simulink model "chapter2_5"  (R2022a)
settings: Solver=FixedStepAuto  StartTime=0.0  StopTime=8  FixedStep=3e-3  RelTol=1e-3
values from neighbouring files (symbols this model uses):
  A = 10    [Figure2_3.m]    (also in parameterLoadForchapter2_4.m = 0.0001; parameterLoadForchapter2_5.m = 0.0001)
  base = Mb*9.8/4+mw*9.8+0.5*9.8*Mv    (= 46305)    [parameterLoadForchapter2_5.m]
  d = 2.6    [parameterLoadForchapter2_4.m]    (also in parameterLoadForchapter2_5.m)
  I = 3055e-8    (= 3.055e-05)    [parameterLoadForchapter2_5.m]
  Jv = 3044    [parameterLoadForchapter2_4.m]    (also in parameterLoadForchapter2_5.m)
  kf = 6.67e7    (= 66700000)    [parameterLoadForchapter2_5.m]
  Kv = 1.5e6    (= 1500000)    [parameterLoadForchapter2_4.m]    (also in parameterLoadForchapter2_5.m)
  L = 15.6    [parameterLoadForchapter2_4.m]    (also in parameterLoadForchapter2_5.m = 210)
  Ln = LNs(i)    [Mchapter2_4.m]    (also in Mchapter2_5.m = LN_set(i))
  m = 7800*(7684e-6)    (= 59.9352)    [parameterLoadForchapter2_5.m]
  Mv = 4060    [parameterLoadForchapter2_4.m]    (also in parameterLoadForchapter2_5.m = 2030)
  mw = 1670    [parameterLoadForchapter2_4.m]    (also in parameterLoadForchapter2_5.m = 835)
  N1 = 0    [Mchapter2_5.m]
  N2 = 0    [Mchapter2_5.m]
  position = 0.5*L    (= 105)    [parameterLoadForchapter2_5.m]
  r = 0.42    [parameterLoadForchapter2_4.m]    (also in parameterLoadForchapter2_5.m)
  R = 400    [Figure2_3.m]    (also in parameterLoadForchapter2_4.m = 0.42; parameterLoadForchapter2_5.m = 0.42)
  v = vkmh/3.6    (= 13.8888888889)    [parameterLoadForchapter2_4.m]    (also in parameterLoadForchapter2_5.m = 27.7777777778)
  vkmh = 50    [parameterLoadForchapter2_4.m]    (also in parameterLoadForchapter2_5.m = 100)
(part 154 of 157)

== system chapter2_5/Subsystem5/Subsystem3/Subsystem6/Subsystem19/Subsystem ==
  In1 : Inport
  k : Inport  Port=2
  In3 : Inport  Port=3
  Add : Sum
  Add1 : Sum  Inputs=-+
  Add2 : Sum  Inputs=+-
  Add3 : Sum  Inputs=+-
  Add4 : Sum  Inputs=+-
  Add5 : Sum  Inputs=-+
  Clock : Clock
  Clock1 : Clock
  Constant : Constant  Value=4
  Constant1 : Constant  Value=kf/m
  Constant2 : Constant
  Constant3 : Constant
  Divide : Product  Inputs=*/
  Divide1 : Product  Inputs=*/
  Divide2 : Product  Inputs=**/
  Gain : Gain  Gain=pi/L
  Gain1 : Gain  Gain=E*I/m
  Gain2 : Gain  Gain=v*pi/L
  Gain3 : Gain  Gain=d/v
  Gain4 : Gain  Gain=-2/(m*L)
  Gain5 : Gain  Gain=pi*position/L
  Power : Math  Operator=pow
  Product{,1..3} (4 alike) : Product
  Sin : Trigonometry
  Sin1 : Trigonometry
  Sin2 : Trigonometry
  Sqrt : Sqrt
  Square : Math  Operator=square
  Square1 : Math  Operator=square
  Out1 : Outport
  tk : Outport  Port=2
  wires:
  [imod] -> Gain/1
  Gain2/1 -> [Omiga_n]
  Gain/1 -> Power/1
  Power/1 -> Gain1/1
  Add/1 -> Sqrt/1
  Gain4/1 -> Divide1/1
  Sin2/1 -> Product2/2
  [Omiga_n] -> Product3/1
  Add3/1 -> Product3/2
  Gain1/1 -> Add/1
  Constant1/1 -> Add/2
  Product3/1 -> Sin1/1
  Sqrt/1 -> [Omiga_bn]
  k/1 -> Add4/1
  Add1/1 -> Divide2/3
  Square/1 -> Add1/1
  Gain5/1 -> Sin/1
  [static_n] -> Product/2
  [Omiga_bn] -> Product1/1
  In1/1 -> [imod]
  Constant2/1 -> Add1/2
  Product/1 -> Divide2/1
  [imod] -> Gain2/1
  Divide/1 -> [Sn]
  [Sn] -> Product2/1
  In3/1 -> Gain4/1
  Divide1/1 -> [static_n]
  [Omiga_bn] -> Square1/1
  [Omiga_n] -> Divide/1
  Square1/1 -> Divide1/2
  [Omiga_bn] -> Divide/2
  [Sn] -> Square/1
  Product2/1 -> Add2/2
  Constant/1 -> Power/2
  [imod] -> Gain5/1
  Sin1/1 -> Add2/1
  Sin/1 -> Product/1
  Product1/1 -> Sin2/1
  Clock/1 -> Add5/2
  Add2/1 -> Divide2/2
  Divide2/1 -> Out1/1
  Clock1/1 -> Add3/1
  Constant3/1 -> Add4/2
  Add4/1 -> Gain3/1
  Gain3/1 -> tk/1, Add5/1, Add3/2
  Add5/1 -> Product1/2

== system chapter2_5/Subsystem5/Subsystem3/Subsystem6/Subsystem19/Subsystem1 ==
  index : Inport
  k : Inport  Port=2
  F : Inport  Port=3
  Add : Sum
  Add1 : Sum  Inputs=-+
  Add2 : Sum  Inputs=+-
  Add3 : Sum  Inputs=+-
  Add4 : Sum  Inputs=+-
  Add5 : Sum  Inputs=-+
  Clock : Clock
  Clock1 : Clock
  Constant : Constant  Value=4
  Constant1 : Constant  Value=kf/m
  Constant2 : Constant
  Constant3 : Constant
  Divide : Product  Inputs=*/
  Divide1 : Product  Inputs=*/
  Divide2 : Product  Inputs=**/
  Gain : Gain  Gain=pi/L
  Gain1 : Gain  Gain=E*I/m
  Gain2 : Gain  Gain=v*pi/L
  Gain3 : Gain  Gain=d/v
  Gain4 : Gain  Gain=-2/(m*L)
  Gain5 : Gain  Gain=pi*position/L
  Power : Math  Operator=pow
  Product{,1..3} (4 alike) : Product
  Sin : Trigonometry
  Sin1 : Trigonometry
  Sin2 : Trigonometry
  Sqrt : Sqrt
  Square : Math  Operator=square
  Square1 : Math  Operator=square
  Out1 : Outport
  tk : Outport  Port=2
  wires:
  Add5/1 -> Product1/2
  Gain3/1 -> Add3/2, Add5/1, tk/1
  Add4/1 -> Gain3/1
  Constant3/1 -> Add4/2
  Clock1/1 -> Add3/1
  Divide2/1 -> Out1/1
  Add2/1 -> Divide2/2
  Clock/1 -> Add5/2
  Product1/1 -> Sin2/1
  Sin/1 -> Product/1
  Sin1/1 -> Add2/1
  [imod] -> Gain5/1
  Constant/1 -> Power/2
  Product2/1 -> Add2/2
  [Sn] -> Square/1
  [Omiga_bn] -> Divide/2
  Square1/1 -> Divide1/2
  [Omiga_n] -> Divide/1
  [Omiga_bn] -> Square1/1
  Divide1/1 -> [static_n]
  F/1 -> Gain4/1
  [Sn] -> Product2/1
  Divide/1 -> [Sn]
  [imod] -> Gain2/1
  Product/1 -> Divide2/1
  Constant2/1 -> Add1/2
  index/1 -> [imod]
  [Omiga_bn] -> Product1/1
  [static_n] -> Product/2
  Gain5/1 -> Sin/1
  Square/1 -> Add1/1
  Add1/1 -> Divide2/3
  k/1 -> Add4/1
  Sqrt/1 -> [Omiga_bn]
  Product3/1 -> Sin1/1
  Constant1/1 -> Add/2
  Gain1/1 -> Add/1
  Add3/1 -> Product3/2
  [Omiga_n] -> Product3/1
  Sin2/1 -> Product2/2
  Gain4/1 -> Divide1/1
  Add/1 -> Sqrt/1
  Power/1 -> Gain1/1
  Gain/1 -> Power/1
  Gain2/1 -> [Omiga_n]
  [imod] -> Gain/1

== system chapter2_5/Subsystem5/Subsystem3/Subsystem6/Subsystem19/Subsystem2 ==
  uxt1 : Inport
  tk : Inport  Port=2
  Add : Sum  Inputs=+-
  Add1 : Sum  Inputs=+--
  Add2 : Sum  Inputs=+-
  C : Constant
  C1 : Constant  Value=0
  C2 : Constant
  C3 : Constant  Value=0
  Clock : Clock
  Clock1 : Clock
  Product : Product
  Switch : Switch  Criteria=u2 > Threshold  SaturateOnIntegerOverflow=off  InputSameDT=off
  Switch1 : Switch  Criteria=u2 > Threshold  SaturateOnIntegerOverflow=off  InputSameDT=off
  deltaT : Constant  Value=L/v
  U1 : Outport
  wires:
  uxt1/1 -> Product/1
  Product/1 -> U1/1
  Clock/1 -> Add/1
  Add/1 -> Switch/2
  C/1 -> Switch/1
  C1/1 -> Switch/3
  C3/1 -> Switch1/3
  C2/1 -> Switch1/1
  Add1/1 -> Switch1/2
  tk/1 -> Add1/2, Add/2
  Clock1/1 -> Add1/1
  deltaT/1 -> Add1/3
  Switch/1 -> Add2/1
  Switch1/1 -> Add2/2
  Add2/1 -> Product/2

== system chapter2_5/Subsystem5/Subsystem3/Subsystem6/Subsystem19/Subsystem3 ==
  uxt1 : Inport
  tk : Inport  Port=2
  Add : Sum  Inputs=+-
  Add1 : Sum  Inputs=+--
  Add2 : Sum  Inputs=+-
  C : Constant
  C1 : Constant  Value=0
  C2 : Constant
  C3 : Constant  Value=0
  Clock : Clock
  Clock1 : Clock
  Product : Product
  Switch : Switch  Criteria=u2 > Threshold  SaturateOnIntegerOverflow=off  InputSameDT=off
  Switch1 : Switch  Criteria=u2 > Threshold  SaturateOnIntegerOverflow=off  InputSameDT=off
  deltaT : Constant  Value=L/v
  U1 : Outport
  wires:
  Add2/1 -> Product/2
  Switch1/1 -> Add2/2
  Switch/1 -> Add2/1
  deltaT/1 -> Add1/3
  Clock1/1 -> Add1/1
  tk/1 -> Add/2, Add1/2
  Add1/1 -> Switch1/2
  C2/1 -> Switch1/1
  C3/1 -> Switch1/3
  C1/1 -> Switch/3
  C/1 -> Switch/1
  Add/1 -> Switch/2
  Clock/1 -> Add/1
  Product/1 -> U1/1
  uxt1/1 -> Product/1

== system chapter2_5/Subsystem5/Subsystem3/Subsystem6/Subsystem2/Subsystem ==
  In1 : Inport
  k : Inport  Port=2
  In3 : Inport  Port=3
  Add : Sum
  Add1 : Sum  Inputs=-+
  Add2 : Sum  Inputs=+-
  Add3 : Sum  Inputs=+-
  Add4 : Sum  Inputs=+-
  Add5 : Sum  Inputs=-+
  Clock : Clock
  Clock1 : Clock
  Constant : Constant  Value=4
  Constant1 : Constant  Value=kf/m
  Constant2 : Constant
  Constant3 : Constant
  Divide : Product  Inputs=*/
  Divide1 : Product  Inputs=*/
  Divide2 : Product  Inputs=**/
  Gain : Gain  Gain=pi/L
  Gain1 : Gain  Gain=E*I/m
  Gain2 : Gain  Gain=v*pi/L
  Gain3 : Gain  Gain=d/v
  Gain4 : Gain  Gain=-2/(m*L)
  Gain5 : Gain  Gain=pi*position/L
  Power : Math  Operator=pow
  Product{,1..3} (4 alike) : Product
  Sin : Trigonometry
  Sin1 : Trigonometry
  Sin2 : Trigonometry
  Sqrt : Sqrt
  Square : Math  Operator=square
  Square1 : Math  Operator=square
  Out1 : Outport
  tk : Outport  Port=2
  wires:
  [imod] -> Gain/1
  Gain2/1 -> [Omiga_n]
  Gain/1 -> Power/1
  Power/1 -> Gain1/1
  Add/1 -> Sqrt/1
  Gain4/1 -> Divide1/1
  Sin2/1 -> Product2/2
  [Omiga_n] -> Product3/1
  Add3/1 -> Product3/2
  Gain1/1 -> Add/1
  Constant1/1 -> Add/2
  Product3/1 -> Sin1/1
  Sqrt/1 -> [Omiga_bn]
  k/1 -> Add4/1
  Add1/1 -> Divide2/3
  Square/1 -> Add1/1
  Gain5/1 -> Sin/1
  [static_n] -> Product/2
  [Omiga_bn] -> Product1/1
  In1/1 -> [imod]
  Constant2/1 -> Add1/2
  Product/1 -> Divide2/1
  [imod] -> Gain2/1
  Divide/1 -> [Sn]
  [Sn] -> Product2/1
  In3/1 -> Gain4/1
  Divide1/1 -> [static_n]
  [Omiga_bn] -> Square1/1
  [Omiga_n] -> Divide/1
  Square1/1 -> Divide1/2
  [Omiga_bn] -> Divide/2
  [Sn] -> Square/1
  Product2/1 -> Add2/2
  Constant/1 -> Power/2
  [imod] -> Gain5/1
  Sin1/1 -> Add2/1
  Sin/1 -> Product/1
  Product1/1 -> Sin2/1
  Clock/1 -> Add5/2
  Add2/1 -> Divide2/2
  Divide2/1 -> Out1/1
  Clock1/1 -> Add3/1
  Constant3/1 -> Add4/2
  Add4/1 -> Gain3/1
  Gain3/1 -> tk/1, Add5/1, Add3/2
  Add5/1 -> Product1/2

== system chapter2_5/Subsystem5/Subsystem3/Subsystem6/Subsystem2/Subsystem1 ==
  index : Inport
  k : Inport  Port=2
  F : Inport  Port=3
  Add : Sum
  Add1 : Sum  Inputs=-+
  Add2 : Sum  Inputs=+-
  Add3 : Sum  Inputs=+-
  Add4 : Sum  Inputs=+-
  Add5 : Sum  Inputs=-+
  Clock : Clock
  Clock1 : Clock
  Constant : Constant  Value=4
  Constant1 : Constant  Value=kf/m
  Constant2 : Constant
  Constant3 : Constant
  Divide : Product  Inputs=*/
  Divide1 : Product  Inputs=*/
  Divide2 : Product  Inputs=**/
  Gain : Gain  Gain=pi/L
  Gain1 : Gain  Gain=E*I/m
  Gain2 : Gain  Gain=v*pi/L
  Gain3 : Gain  Gain=d/v
  Gain4 : Gain  Gain=-2/(m*L)
  Gain5 : Gain  Gain=pi*position/L
  Power : Math  Operator=pow
  Product{,1..3} (4 alike) : Product
  Sin : Trigonometry
  Sin1 : Trigonometry
  Sin2 : Trigonometry
  Sqrt : Sqrt
  Square : Math  Operator=square
  Square1 : Math  Operator=square
  Out1 : Outport
  tk : Outport  Port=2
  wires:
  Add5/1 -> Product1/2
  Gain3/1 -> Add3/2, Add5/1, tk/1
  Add4/1 -> Gain3/1
  Constant3/1 -> Add4/2
  Clock1/1 -> Add3/1
  Divide2/1 -> Out1/1
  Add2/1 -> Divide2/2
  Clock/1 -> Add5/2
  Product1/1 -> Sin2/1
  Sin/1 -> Product/1
  Sin1/1 -> Add2/1
  [imod] -> Gain5/1
  Constant/1 -> Power/2
  Product2/1 -> Add2/2
  [Sn] -> Square/1
  [Omiga_bn] -> Divide/2
  Square1/1 -> Divide1/2
  [Omiga_n] -> Divide/1
  [Omiga_bn] -> Square1/1
  Divide1/1 -> [static_n]
  F/1 -> Gain4/1
  [Sn] -> Product2/1
  Divide/1 -> [Sn]
  [imod] -> Gain2/1
  Product/1 -> Divide2/1
  Constant2/1 -> Add1/2
  index/1 -> [imod]
  [Omiga_bn] -> Product1/1
  [static_n] -> Product/2
  Gain5/1 -> Sin/1
  Square/1 -> Add1/1
  Add1/1 -> Divide2/3
  k/1 -> Add4/1
  Sqrt/1 -> [Omiga_bn]
  Product3/1 -> Sin1/1
  Constant1/1 -> Add/2
  Gain1/1 -> Add/1
  Add3/1 -> Product3/2
  [Omiga_n] -> Product3/1
  Sin2/1 -> Product2/2
  Gain4/1 -> Divide1/1
  Add/1 -> Sqrt/1
  Power/1 -> Gain1/1
  Gain/1 -> Power/1
  Gain2/1 -> [Omiga_n]
  [imod] -> Gain/1

== system chapter2_5/Subsystem5/Subsystem3/Subsystem6/Subsystem2/Subsystem2 ==
  uxt1 : Inport
  tk : Inport  Port=2
  Add : Sum  Inputs=+-
  Add1 : Sum  Inputs=+--
  Add2 : Sum  Inputs=+-
  C : Constant
  C1 : Constant  Value=0
  C2 : Constant
  C3 : Constant  Value=0
  Clock : Clock
  Clock1 : Clock
  Product : Product
  Switch : Switch  Criteria=u2 > Threshold  SaturateOnIntegerOverflow=off  InputSameDT=off
  Switch1 : Switch  Criteria=u2 > Threshold  SaturateOnIntegerOverflow=off  InputSameDT=off
  deltaT : Constant  Value=L/v
  U1 : Outport
  wires:
  uxt1/1 -> Product/1
  Product/1 -> U1/1
  Clock/1 -> Add/1
  Add/1 -> Switch/2
  C/1 -> Switch/1
  C1/1 -> Switch/3
  C3/1 -> Switch1/3
  C2/1 -> Switch1/1
  Add1/1 -> Switch1/2
  tk/1 -> Add1/2, Add/2
  Clock1/1 -> Add1/1
  deltaT/1 -> Add1/3
  Switch/1 -> Add2/1
  Switch1/1 -> Add2/2
  Add2/1 -> Product/2

== system chapter2_5/Subsystem5/Subsystem3/Subsystem6/Subsystem2/Subsystem3 ==
  uxt1 : Inport
  tk : Inport  Port=2
  Add : Sum  Inputs=+-
  Add1 : Sum  Inputs=+--
  Add2 : Sum  Inputs=+-
  C : Constant
  C1 : Constant  Value=0
  C2 : Constant
  C3 : Constant  Value=0
  Clock : Clock
  Clock1 : Clock
  Product : Product
  Switch : Switch  Criteria=u2 > Threshold  SaturateOnIntegerOverflow=off  InputSameDT=off
  Switch1 : Switch  Criteria=u2 > Threshold  SaturateOnIntegerOverflow=off  InputSameDT=off
  deltaT : Constant  Value=L/v
  U1 : Outport
  wires:
  Add2/1 -> Product/2
  Switch1/1 -> Add2/2
  Switch/1 -> Add2/1
  deltaT/1 -> Add1/3
  Clock1/1 -> Add1/1
  tk/1 -> Add/2, Add1/2
  Add1/1 -> Switch1/2
  C2/1 -> Switch1/1
  C3/1 -> Switch1/3
  C1/1 -> Switch/3
  C/1 -> Switch/1
  Add/1 -> Switch/2
  Clock/1 -> Add/1
  Product/1 -> U1/1
  uxt1/1 -> Product/1

== system chapter2_5/Subsystem5/Subsystem3/Subsystem6/Subsystem21/Subsystem ==
  In1 : Inport
  k : Inport  Port=2
  In3 : Inport  Port=3
  Add : Sum
  Add1 : Sum  Inputs=-+
  Add2 : Sum  Inputs=+-
  Add3 : Sum  Inputs=+-
  Add4 : Sum  Inputs=+-
  Add5 : Sum  Inputs=-+
  Clock : Clock
  Clock1 : Clock
  Constant : Constant  Value=4
  Constant1 : Constant  Value=kf/m
  Constant2 : Constant
  Constant3 : Constant
  Divide : Product  Inputs=*/
  Divide1 : Product  Inputs=*/
  Divide2 : Product  Inputs=**/
  Gain : Gain  Gain=pi/L
  Gain1 : Gain  Gain=E*I/m
  Gain2 : Gain  Gain=v*pi/L
  Gain3 : Gain  Gain=d/v
  Gain4 : Gain  Gain=-2/(m*L)
  Gain5 : Gain  Gain=pi*position/L
  Power : Math  Operator=pow
  Product{,1..3} (4 alike) : Product
  Sin : Trigonometry
  Sin1 : Trigonometry
  Sin2 : Trigonometry
  Sqrt : Sqrt
  Square : Math  Operator=square
  Square1 : Math  Operator=square
  Out1 : Outport
  tk : Outport  Port=2
  wires:
  [imod] -> Gain/1
  Gain2/1 -> [Omiga_n]
  Gain/1 -> Power/1
  Power/1 -> Gain1/1
  Add/1 -> Sqrt/1
  Gain4/1 -> Divide1/1
  Sin2/1 -> Product2/2
  [Omiga_n] -> Product3/1
  Add3/1 -> Product3/2
  Gain1/1 -> Add/1
  Constant1/1 -> Add/2
  Product3/1 -> Sin1/1
  Sqrt/1 -> [Omiga_bn]
  k/1 -> Add4/1
  Add1/1 -> Divide2/3
  Square/1 -> Add1/1
  Gain5/1 -> Sin/1
  [static_n] -> Product/2
  [Omiga_bn] -> Product1/1
  In1/1 -> [imod]
  Constant2/1 -> Add1/2
  Product/1 -> Divide2/1
  [imod] -> Gain2/1
  Divide/1 -> [Sn]
  [Sn] -> Product2/1
  In3/1 -> Gain4/1
  Divide1/1 -> [static_n]
  [Omiga_bn] -> Square1/1
  [Omiga_n] -> Divide/1
  Square1/1 -> Divide1/2
  [Omiga_bn] -> Divide/2
  [Sn] -> Square/1
  Product2/1 -> Add2/2
  Constant/1 -> Power/2
  [imod] -> Gain5/1
  Sin1/1 -> Add2/1
  Sin/1 -> Product/1
  Product1/1 -> Sin2/1
  Clock/1 -> Add5/2
  Add2/1 -> Divide2/2
  Divide2/1 -> Out1/1
  Clock1/1 -> Add3/1
  Constant3/1 -> Add4/2
  Add4/1 -> Gain3/1
  Gain3/1 -> tk/1, Add5/1, Add3/2
  Add5/1 -> Product1/2

== system chapter2_5/Subsystem5/Subsystem3/Subsystem6/Subsystem21/Subsystem1 ==
  index : Inport
  k : Inport  Port=2
  F : Inport  Port=3
  Add : Sum
  Add1 : Sum  Inputs=-+
  Add2 : Sum  Inputs=+-
  Add3 : Sum  Inputs=+-
  Add4 : Sum  Inputs=+-
  Add5 : Sum  Inputs=-+
  Clock : Clock
  Clock1 : Clock
  Constant : Constant  Value=4
  Constant1 : Constant  Value=kf/m
  Constant2 : Constant
  Constant3 : Constant
  Divide : Product  Inputs=*/
  Divide1 : Product  Inputs=*/
  Divide2 : Product  Inputs=**/
  Gain : Gain  Gain=pi/L
  Gain1 : Gain  Gain=E*I/m
  Gain2 : Gain  Gain=v*pi/L
  Gain3 : Gain  Gain=d/v
  Gain4 : Gain  Gain=-2/(m*L)
  Gain5 : Gain  Gain=pi*position/L
  Power : Math  Operator=pow
  Product{,1..3} (4 alike) : Product
  Sin : Trigonometry
  Sin1 : Trigonometry
  Sin2 : Trigonometry
  Sqrt : Sqrt
  Square : Math  Operator=square
  Square1 : Math  Operator=square
  Out1 : Outport
  tk : Outport  Port=2
  wires:
  Add5/1 -> Product1/2
  Gain3/1 -> Add3/2, Add5/1, tk/1
  Add4/1 -> Gain3/1
  Constant3/1 -> Add4/2
  Clock1/1 -> Add3/1
  Divide2/1 -> Out1/1
  Add2/1 -> Divide2/2
  Clock/1 -> Add5/2
  Product1/1 -> Sin2/1
  Sin/1 -> Product/1
  Sin1/1 -> Add2/1
  [imod] -> Gain5/1
  Constant/1 -> Power/2
  Product2/1 -> Add2/2
  [Sn] -> Square/1
  [Omiga_bn] -> Divide/2
  Square1/1 -> Divide1/2
  [Omiga_n] -> Divide/1
  [Omiga_bn] -> Square1/1
  Divide1/1 -> [static_n]
  F/1 -> Gain4/1
  [Sn] -> Product2/1
  Divide/1 -> [Sn]
  [imod] -> Gain2/1
  Product/1 -> Divide2/1
  Constant2/1 -> Add1/2
  index/1 -> [imod]
  [Omiga_bn] -> Product1/1
  [static_n] -> Product/2
  Gain5/1 -> Sin/1
  Square/1 -> Add1/1
  Add1/1 -> Divide2/3
  k/1 -> Add4/1
  Sqrt/1 -> [Omiga_bn]
  Product3/1 -> Sin1/1
  Constant1/1 -> Add/2
  Gain1/1 -> Add/1
  Add3/1 -> Product3/2
  [Omiga_n] -> Product3/1
  Sin2/1 -> Product2/2
  Gain4/1 -> Divide1/1
  Add/1 -> Sqrt/1
  Power/1 -> Gain1/1
  Gain/1 -> Power/1
  Gain2/1 -> [Omiga_n]
  [imod] -> Gain/1

== system chapter2_5/Subsystem5/Subsystem3/Subsystem6/Subsystem21/Subsystem2 ==
  uxt1 : Inport
  tk : Inport  Port=2
  Add : Sum  Inputs=+-
  Add1 : Sum  Inputs=+--
  Add2 : Sum  Inputs=+-
  C : Constant
  C1 : Constant  Value=0
  C2 : Constant
  C3 : Constant  Value=0
  Clock : Clock
  Clock1 : Clock
  Product : Product
  Switch : Switch  Criteria=u2 > Threshold  SaturateOnIntegerOverflow=off  InputSameDT=off
  Switch1 : Switch  Criteria=u2 > Threshold  SaturateOnIntegerOverflow=off  InputSameDT=off
  deltaT : Constant  Value=L/v
  U1 : Outport
  wires:
  uxt1/1 -> Product/1
  Product/1 -> U1/1
  Clock/1 -> Add/1
  Add/1 -> Switch/2
  C/1 -> Switch/1
  C1/1 -> Switch/3
  C3/1 -> Switch1/3
  C2/1 -> Switch1/1
  Add1/1 -> Switch1/2
  tk/1 -> Add1/2, Add/2
  Clock1/1 -> Add1/1
  deltaT/1 -> Add1/3
  Switch/1 -> Add2/1
  Switch1/1 -> Add2/2
  Add2/1 -> Product/2

== system chapter2_5/Subsystem5/Subsystem3/Subsystem6/Subsystem21/Subsystem3 ==
  uxt1 : Inport
  tk : Inport  Port=2
  Add : Sum  Inputs=+-
  Add1 : Sum  Inputs=+--
  Add2 : Sum  Inputs=+-
  C : Constant
  C1 : Constant  Value=0
  C2 : Constant
  C3 : Constant  Value=0
  Clock : Clock
  Clock1 : Clock
  Product : Product
  Switch : Switch  Criteria=u2 > Threshold  SaturateOnIntegerOverflow=off  InputSameDT=off
  Switch1 : Switch  Criteria=u2 > Threshold  SaturateOnIntegerOverflow=off  InputSameDT=off
  deltaT : Constant  Value=L/v
  U1 : Outport
  wires:
  Add2/1 -> Product/2
  Switch1/1 -> Add2/2
  Switch/1 -> Add2/1
  deltaT/1 -> Add1/3
  Clock1/1 -> Add1/1
  tk/1 -> Add/2, Add1/2
  Add1/1 -> Switch1/2
  C2/1 -> Switch1/1
  C3/1 -> Switch1/3
  C1/1 -> Switch/3
  C/1 -> Switch/1
  Add/1 -> Switch/2
  Clock/1 -> Add/1
  Product/1 -> U1/1
  uxt1/1 -> Product/1

== system chapter2_5/Subsystem5/Subsystem3/Subsystem6/Subsystem3/Subsystem ==
  In1 : Inport
  k : Inport  Port=2
  In3 : Inport  Port=3
  Add : Sum
  Add1 : Sum  Inputs=-+
  Add2 : Sum  Inputs=+-
  Add3 : Sum  Inputs=+-
  Add4 : Sum  Inputs=+-
  Add5 : Sum  Inputs=-+
  Clock : Clock
  Clock1 : Clock
  Constant : Constant  Value=4
  Constant1 : Constant  Value=kf/m
  Constant2 : Constant
  Constant3 : Constant
  Divide : Product  Inputs=*/
  Divide1 : Product  Inputs=*/
  Divide2 : Product  Inputs=**/
  Gain : Gain  Gain=pi/L
  Gain1 : Gain  Gain=E*I/m
  Gain2 : Gain  Gain=v*pi/L
  Gain3 : Gain  Gain=d/v
  Gain4 : Gain  Gain=-2/(m*L)
  Gain5 : Gain  Gain=pi*position/L
  Power : Math  Operator=pow
  Product{,1..3} (4 alike) : Product
  Sin : Trigonometry
  Sin1 : Trigonometry
  Sin2 : Trigonometry
  Sqrt : Sqrt
  Square : Math  Operator=square
  Square1 : Math  Operator=square
  Out1 : Outport
  tk : Outport  Port=2
  wires:
  Add5/1 -> Product1/2
  Gain3/1 -> Add3/2, Add5/1, tk/1
  Add4/1 -> Gain3/1
  Constant3/1 -> Add4/2
  Clock1/1 -> Add3/1
  Divide2/1 -> Out1/1
  Add2/1 -> Divide2/2
  Clock/1 -> Add5/2
  Product1/1 -> Sin2/1
  Sin/1 -> Product/1
  Sin1/1 -> Add2/1
  [imod] -> Gain5/1
  Constant/1 -> Power/2
  Product2/1 -> Add2/2
  [Sn] -> Square/1
  [Omiga_bn] -> Divide/2
  Square1/1 -> Divide1/2
  [Omiga_n] -> Divide/1
  [Omiga_bn] -> Square1/1
  Divide1/1 -> [static_n]
  In3/1 -> Gain4/1
  [Sn] -> Product2/1
  Divide/1 -> [Sn]
  [imod] -> Gain2/1
  Product/1 -> Divide2/1
  Constant2/1 -> Add1/2
  In1/1 -> [imod]
  [Omiga_bn] -> Product1/1
  [static_n] -> Product/2
  Gain5/1 -> Sin/1
  Square/1 -> Add1/1
  Add1/1 -> Divide2/3
  k/1 -> Add4/1
  Sqrt/1 -> [Omiga_bn]
  Product3/1 -> Sin1/1
  Constant1/1 -> Add/2
  Gain1/1 -> Add/1
  Add3/1 -> Product3/2
  [Omiga_n] -> Product3/1
  Sin2/1 -> Product2/2
  Gain4/1 -> Divide1/1
  Add/1 -> Sqrt/1
  Power/1 -> Gain1/1
  Gain/1 -> Power/1
  Gain2/1 -> [Omiga_n]
  [imod] -> Gain/1

== system chapter2_5/Subsystem5/Subsystem3/Subsystem6/Subsystem3/Subsystem1 ==
  index : Inport
  k : Inport  Port=2
  F : Inport  Port=3
  Add : Sum
  Add1 : Sum  Inputs=-+
  Add2 : Sum  Inputs=+-
  Add3 : Sum  Inputs=+-
  Add4 : Sum  Inputs=+-
  Add5 : Sum  Inputs=-+
  Clock : Clock
  Clock1 : Clock
  Constant : Constant  Value=4
  Constant1 : Constant  Value=kf/m
  Constant2 : Constant
  Constant3 : Constant
  Divide : Product  Inputs=*/
  Divide1 : Product  Inputs=*/
  Divide2 : Product  Inputs=**/
  Gain : Gain  Gain=pi/L
  Gain1 : Gain  Gain=E*I/m
  Gain2 : Gain  Gain=v*pi/L
  Gain3 : Gain  Gain=d/v
  Gain4 : Gain  Gain=-2/(m*L)
  Gain5 : Gain  Gain=pi*position/L
  Power : Math  Operator=pow
  Product{,1..3} (4 alike) : Product
  Sin : Trigonometry
  Sin1 : Trigonometry
  Sin2 : Trigonometry
  Sqrt : Sqrt
  Square : Math  Operator=square
  Square1 : Math  Operator=square
  Out1 : Outport
  tk : Outport  Port=2
  wires:
  [imod] -> Gain/1
  Gain2/1 -> [Omiga_n]
  Gain/1 -> Power/1
  Power/1 -> Gain1/1
  Add/1 -> Sqrt/1
  Gain4/1 -> Divide1/1
  Sin2/1 -> Product2/2
  [Omiga_n] -> Product3/1
  Add3/1 -> Product3/2
  Gain1/1 -> Add/1
  Constant1/1 -> Add/2
  Product3/1 -> Sin1/1
  Sqrt/1 -> [Omiga_bn]
  k/1 -> Add4/1
  Add1/1 -> Divide2/3
  Square/1 -> Add1/1
  Gain5/1 -> Sin/1
  [static_n] -> Product/2
  [Omiga_bn] -> Product1/1
  index/1 -> [imod]
  Constant2/1 -> Add1/2
  Product/1 -> Divide2/1
  [imod] -> Gain2/1
  Divide/1 -> [Sn]
  [Sn] -> Product2/1
  F/1 -> Gain4/1
  Divide1/1 -> [static_n]
  [Omiga_bn] -> Square1/1
  [Omiga_n] -> Divide/1
  Square1/1 -> Divide1/2
  [Omiga_bn] -> Divide/2
  [Sn] -> Square/1
  Product2/1 -> Add2/2
  Constant/1 -> Power/2
  [imod] -> Gain5/1
  Sin1/1 -> Add2/1
  Sin/1 -> Product/1
  Product1/1 -> Sin2/1
  Clock/1 -> Add5/2
  Add2/1 -> Divide2/2
  Divide2/1 -> Out1/1
  Clock1/1 -> Add3/1
  Constant3/1 -> Add4/2
  Add4/1 -> Gain3/1
  Gain3/1 -> tk/1, Add5/1, Add3/2
  Add5/1 -> Product1/2

== system chapter2_5/Subsystem5/Subsystem3/Subsystem6/Subsystem3/Subsystem2 ==
  uxt1 : Inport
  tk : Inport  Port=2
  Add : Sum  Inputs=+-
  Add1 : Sum  Inputs=+--
  Add2 : Sum  Inputs=+-
  C : Constant
  C1 : Constant  Value=0
  C2 : Constant
  C3 : Constant  Value=0
  Clock : Clock
  Clock1 : Clock
  Product : Product
  Switch : Switch  Criteria=u2 > Threshold  SaturateOnIntegerOverflow=off  InputSameDT=off
  Switch1 : Switch  Criteria=u2 > Threshold  SaturateOnIntegerOverflow=off  InputSameDT=off
  deltaT : Constant  Value=L/v
  U1 : Outport
  wires:
  Add2/1 -> Product/2
  Switch1/1 -> Add2/2
  Switch/1 -> Add2/1
  deltaT/1 -> Add1/3
  Clock1/1 -> Add1/1
  tk/1 -> Add/2, Add1/2
  Add1/1 -> Switch1/2
  C2/1 -> Switch1/1
  C3/1 -> Switch1/3
  C1/1 -> Switch/3
  C/1 -> Switch/1
  Add/1 -> Switch/2
  Clock/1 -> Add/1
  Product/1 -> U1/1
  uxt1/1 -> Product/1

== system chapter2_5/Subsystem5/Subsystem3/Subsystem6/Subsystem3/Subsystem3 ==
  uxt1 : Inport
  tk : Inport  Port=2
  Add : Sum  Inputs=+-
  Add1 : Sum  Inputs=+--
  Add2 : Sum  Inputs=+-
  C : Constant
  C1 : Constant  Value=0
  C2 : Constant
  C3 : Constant  Value=0
  Clock : Clock
  Clock1 : Clock
  Product : Product
  Switch : Switch  Criteria=u2 > Threshold  SaturateOnIntegerOverflow=off  InputSameDT=off
  Switch1 : Switch  Criteria=u2 > Threshold  SaturateOnIntegerOverflow=off  InputSameDT=off
  deltaT : Constant  Value=L/v
  U1 : Outport
  wires:
  uxt1/1 -> Product/1
  Product/1 -> U1/1
  Clock/1 -> Add/1
  Add/1 -> Switch/2
  C/1 -> Switch/1
  C1/1 -> Switch/3
  C3/1 -> Switch1/3
  C2/1 -> Switch1/1
  Add1/1 -> Switch1/2
  tk/1 -> Add1/2, Add/2
  Clock1/1 -> Add1/1
  deltaT/1 -> Add1/3
  Switch/1 -> Add2/1
  Switch1/1 -> Add2/2
  Add2/1 -> Product/2
